simulink model "doa_debug2"  (R2013b) (continued)
== system doa_debug2 == (continued)
      | port_label('output', 1, 'q');
      | disp(['Z^-', num2str(latency)]);
  pipeline91 : Subsystem  AncestorBlock=casper_library_delays/pipeline  mask(pipeline){latency=1}
      mask help:
      | An explicitly laid-out delay line to avoid mapping to SRL16s
      | for use in pipelining to help achieve timing closure.
      mask init:
      | pipeline_init(gcb,'latency',latency);
      settings: AttributesFormatString=Latency = %<latency>
      mask Display:
      | port_label('input', 1, 'd');
      | port_label('output', 1, 'q');
      | disp(['Z^-', num2str(latency)]);
  pipeline92 : Subsystem  AncestorBlock=casper_library_delays/pipeline  mask(pipeline){latency=2}
      mask help:
      | An explicitly laid-out delay line to avoid mapping to SRL16s
      | for use in pipelining to help achieve timing closure.
      mask init:
      | pipeline_init(gcb,'latency',latency);
      settings: AttributesFormatString=Latency = %<latency>
      mask Display:
      | port_label('input', 1, 'd');
      | port_label('output', 1, 'q');
      | disp(['Z^-', num2str(latency)]);
  pipeline97 : Subsystem  AncestorBlock=casper_library_delays/pipeline  mask(pipeline){latency=2}
      mask help:
      | An explicitly laid-out delay line to avoid mapping to SRL16s
      | for use in pipelining to help achieve timing closure.
      mask init:
      | pipeline_init(gcb,'latency',latency);
      settings: AttributesFormatString=Latency = %<latency>
      mask Display:
      | port_label('input', 1, 'd');
      | port_label('output', 1, 'q');
      | disp(['Z^-', num2str(latency)]);
  pipeline98 : Subsystem  AncestorBlock=casper_library_delays/pipeline  mask(pipeline){latency=1}
      mask help:
      | An explicitly laid-out delay line to avoid mapping to SRL16s
      | for use in pipelining to help achieve timing closure.
      mask init:
      | pipeline_init(gcb,'latency',latency);
      settings: AttributesFormatString=Latency = %<latency>
      mask Display:
      | port_label('input', 1, 'd');
      | port_label('output', 1, 'q');
      | disp(['Z^-', num2str(latency)]);
  power{,1..7} (8 alike) : casper_library_misc/power  BitWidth=18  add_latency=2  mult_latency=3
  r11_0 : Subsystem  Tag=xps:bram  AncestorBlock=xps_library/Shared_BRAM  mask{arith_type=Unsigned, addr_width=11, data_width=16, reg_prim_output=off, reg_core_output=off, optimization=Minimum_Area, data_bin_pt=0, init_vals=[0:2^10-1], sample_rate=1}
      mask prompts: arith_type "Output Data Type"; addr_width "Address width"; data_width "Data Width"; reg_prim_output "Register Primitive Output"; reg_core_output "Register Core Output"; data_bin_pt "Data Binary Point"; init_vals "Initial values (simulation only)"; sample_rate "Sample rate"
      mask init:
      | shared_bram_mask;
      mask TypeOptions arith_type: Unsigned | Signed  (2's comp)
      mask TypeOptions data_width: 8 | 16 | 32 | 64 | 128
      mask TypeOptions optimization: Minimum_Area | Low_Power
  r11_1 : Subsystem  Tag=xps:bram  AncestorBlock=xps_library/Shared_BRAM  mask{arith_type=Unsigned, addr_width=11, data_width=16, reg_prim_output=off, reg_core_output=off, optimization=Minimum_Area, data_bin_pt=0, init_vals=[0:2^10-1], sample_rate=1}
      mask prompts: arith_type "Output Data Type"; addr_width "Address width"; data_width "Data Width"; reg_prim_output "Register Primitive Output"; reg_core_output "Register Core Output"; data_bin_pt "Data Binary Point"; init_vals "Initial values (simulation only)"; sample_rate "Sample rate"
      mask init:
      | shared_bram_mask;
      mask TypeOptions arith_type: Unsigned | Signed  (2's comp)
      mask TypeOptions data_width: 8 | 16 | 32 | 64 | 128
      mask TypeOptions optimization: Minimum_Area | Low_Power
  r11_2 : Subsystem  Tag=xps:bram  AncestorBlock=xps_library/Shared_BRAM  mask{arith_type=Unsigned, addr_width=11, data_width=16, reg_prim_output=off, reg_core_output=off, optimization=Minimum_Area, data_bin_pt=0, init_vals=[0:2^10-1], sample_rate=1}
      mask prompts: arith_type "Output Data Type"; addr_width "Address width"; data_width "Data Width"; reg_prim_output "Register Primitive Output"; reg_core_output "Register Core Output"; data_bin_pt "Data Binary Point"; init_vals "Initial values (simulation only)"; sample_rate "Sample rate"
      mask init:
      | shared_bram_mask;
      mask TypeOptions arith_type: Unsigned | Signed  (2's comp)
      mask TypeOptions data_width: 8 | 16 | 32 | 64 | 128
      mask TypeOptions optimization: Minimum_Area | Low_Power
  r11_3 : Subsystem  Tag=xps:bram  AncestorBlock=xps_library/Shared_BRAM  mask{arith_type=Unsigned, addr_width=11, data_width=16, reg_prim_output=off, reg_core_output=off, optimization=Minimum_Area, data_bin_pt=0, init_vals=[0:2^10-1], sample_rate=1}
      mask prompts: arith_type "Output Data Type"; addr_width "Address width"; data_width "Data Width"; reg_prim_output "Register Primitive Output"; reg_core_output "Register Core Output"; data_bin_pt "Data Binary Point"; init_vals "Initial values (simulation only)"; sample_rate "Sample rate"
      mask init:
      | shared_bram_mask;
      mask TypeOptions arith_type: Unsigned | Signed  (2's comp)
      mask TypeOptions data_width: 8 | 16 | 32 | 64 | 128
      mask TypeOptions optimization: Minimum_Area | Low_Power
  r12_0 : Subsystem  Tag=xps:bram  AncestorBlock=xps_library/Shared_BRAM  mask{arith_type=Unsigned, addr_width=11, data_width=16, reg_prim_output=off, reg_core_output=off, optimization=Minimum_Area, data_bin_pt=0, init_vals=[0:2^10-1], sample_rate=1}
      mask prompts: arith_type "Output Data Type"; addr_width "Address width"; data_width "Data Width"; reg_prim_output "Register Primitive Output"; reg_core_output "Register Core Output"; data_bin_pt "Data Binary Point"; init_vals "Initial values (simulation only)"; sample_rate "Sample rate"
      mask init:
      | shared_bram_mask;
      mask TypeOptions arith_type: Unsigned | Signed  (2's comp)
      mask TypeOptions data_width: 8 | 16 | 32 | 64 | 128
      mask TypeOptions optimization: Minimum_Area | Low_Power
  r12_1 : Subsystem  Tag=xps:bram  AncestorBlock=xps_library/Shared_BRAM  mask{arith_type=Unsigned, addr_width=11, data_width=16, reg_prim_output=off, reg_core_output=off, optimization=Minimum_Area, data_bin_pt=0, init_vals=[0:2^10-1], sample_rate=1}
      mask prompts: arith_type "Output Data Type"; addr_width "Address width"; data_width "Data Width"; reg_prim_output "Register Primitive Output"; reg_core_output "Register Core Output"; data_bin_pt "Data Binary Point"; init_vals "Initial values (simulation only)"; sample_rate "Sample rate"
      mask init:
      | shared_bram_mask;
      mask TypeOptions arith_type: Unsigned | Signed  (2's comp)
      mask TypeOptions data_width: 8 | 16 | 32 | 64 | 128
      mask TypeOptions optimization: Minimum_Area | Low_Power
  r12_2 : Subsystem  Tag=xps:bram  AncestorBlock=xps_library/Shared_BRAM  mask{arith_type=Unsigned, addr_width=11, data_width=16, reg_prim_output=off, reg_core_output=off, optimization=Minimum_Area, data_bin_pt=0, init_vals=[0:2^10-1], sample_rate=1}
      mask prompts: arith_type "Output Data Type"; addr_width "Address width"; data_width "Data Width"; reg_prim_output "Register Primitive Output"; reg_core_output "Register Core Output"; data_bin_pt "Data Binary Point"; init_vals "Initial values (simulation only)"; sample_rate "Sample rate"
      mask init:
      | shared_bram_mask;
      mask TypeOptions arith_type: Unsigned | Signed  (2's comp)
      mask TypeOptions data_width: 8 | 16 | 32 | 64 | 128
      mask TypeOptions optimization: Minimum_Area | Low_Power
  r12_3 : Subsystem  Tag=xps:bram  AncestorBlock=xps_library/Shared_BRAM  mask{arith_type=Unsigned, addr_width=11, data_width=16, reg_prim_output=off, reg_core_output=off, optimization=Minimum_Area, data_bin_pt=0, init_vals=[0:2^10-1], sample_rate=1}
      mask prompts: arith_type "Output Data Type"; addr_width "Address width"; data_width "Data Width"; reg_prim_output "Register Primitive Output"; reg_core_output "Register Core Output"; data_bin_pt "Data Binary Point"; init_vals "Initial values (simulation only)"; sample_rate "Sample rate"
      mask init:
      | shared_bram_mask;
      mask TypeOptions arith_type: Unsigned | Signed  (2's comp)
      mask TypeOptions data_width: 8 | 16 | 32 | 64 | 128
      mask TypeOptions optimization: Minimum_Area | Low_Power
  r22_0 : Subsystem  Tag=xps:bram  AncestorBlock=xps_library/Shared_BRAM  mask{arith_type=Unsigned, addr_width=11, data_width=16, reg_prim_output=off, reg_core_output=off, optimization=Minimum_Area, data_bin_pt=0, init_vals=[0:2^10-1], sample_rate=1}
      mask prompts: arith_type "Output Data Type"; addr_width "Address width"; data_width "Data Width"; reg_prim_output "Register Primitive Output"; reg_core_output "Register Core Output"; data_bin_pt "Data Binary Point"; init_vals "Initial values (simulation only)"; sample_rate "Sample rate"
      mask init:
      | shared_bram_mask;
      mask TypeOptions arith_type: Unsigned | Signed  (2's comp)
      mask TypeOptions data_width: 8 | 16 | 32 | 64 | 128
      mask TypeOptions optimization: Minimum_Area | Low_Power
  r22_1 : Subsystem  Tag=xps:bram  AncestorBlock=xps_library/Shared_BRAM  mask{arith_type=Unsigned, addr_width=11, data_width=16, reg_prim_output=off, reg_core_output=off, optimization=Minimum_Area, data_bin_pt=0, init_vals=[0:2^10-1], sample_rate=1}
      mask prompts: arith_type "Output Data Type"; addr_width "Address width"; data_width "Data Width"; reg_prim_output "Register Primitive Output"; reg_core_output "Register Core Output"; data_bin_pt "Data Binary Point"; init_vals "Initial values (simulation only)"; sample_rate "Sample rate"
      mask init:
      | shared_bram_mask;
      mask TypeOptions arith_type: Unsigned | Signed  (2's comp)
      mask TypeOptions data_width: 8 | 16 | 32 | 64 | 128
      mask TypeOptions optimization: Minimum_Area | Low_Power
  r22_2 : Subsystem  Tag=xps:bram  AncestorBlock=xps_library/Shared_BRAM  mask{arith_type=Unsigned, addr_width=11, data_width=16, reg_prim_output=off, reg_core_output=off, optimization=Minimum_Area, data_bin_pt=0, init_vals=[0:2^10-1], sample_rate=1}
      mask prompts: arith_type "Output Data Type"; addr_width "Address width"; data_width "Data Width"; reg_prim_output "Register Primitive Output"; reg_core_output "Register Core Output"; data_bin_pt "Data Binary Point"; init_vals "Initial values (simulation only)"; sample_rate "Sample rate"
      mask init:
      | shared_bram_mask;
      mask TypeOptions arith_type: Unsigned | Signed  (2's comp)
      mask TypeOptions data_width: 8 | 16 | 32 | 64 | 128
      mask TypeOptions optimization: Minimum_Area | Low_Power
  r22_3 : Subsystem  Tag=xps:bram  AncestorBlock=xps_library/Shared_BRAM  mask{arith_type=Unsigned, addr_width=11, data_width=16, reg_prim_output=off, reg_core_output=off, optimization=Minimum_Area, data_bin_pt=0, init_vals=[0:2^10-1], sample_rate=1}
      mask prompts: arith_type "Output Data Type"; addr_width "Address width"; data_width "Data Width"; reg_prim_output "Register Primitive Output"; reg_core_output "Register Core Output"; data_bin_pt "Data Binary Point"; init_vals "Initial values (simulation only)"; sample_rate "Sample rate"
      mask init:
      | shared_bram_mask;
      mask TypeOptions arith_type: Unsigned | Signed  (2's comp)
      mask TypeOptions data_width: 8 | 16 | 32 | 64 | 128
      mask TypeOptions optimization: Minimum_Area | Low_Power
  simple_bram_vacc_0a_0 : Subsystem  AncestorBlock=casper_library_accumulators/simple_bram_vacc  mask(simple_bram_vacc){vec_len=512, arith_type=Unsigned, n_bits=64, bin_pt=35}
      mask prompts: vec_len "Vector length:"; arith_type "Output type:"; n_bits "Bit width (output):"; bin_pt "Binary point (output):"
      mask help: This simple vector accumulator is based on a shift register. It outputs its previous accumulated vector after a new_acc pulse is received.
      mask init:
      | simple_bram_vacc_init(gcb, ...
      |     'vec_len', vec_len, ...
      |     'arith_type', arith_type, ...
      |     'n_bits', n_bits, ...
      |     'bin_pt', bin_pt);
      mask TypeOptions arith_type: Signed | Unsigned
  simple_bram_vacc_0a_1 : Subsystem  AncestorBlock=casper_library_accumulators/simple_bram_vacc  mask(simple_bram_vacc){vec_len=512, arith_type=Unsigned, n_bits=64, bin_pt=35}
      mask prompts: vec_len "Vector length:"; arith_type "Output type:"; n_bits "Bit width (output):"; bin_pt "Binary point (output):"
      mask help: This simple vector accumulator is based on a shift register. It outputs its previous accumulated vector after a new_acc pulse is received.
      mask init:
      | simple_bram_vacc_init(gcb, ...
      |     'vec_len', vec_len, ...
      |     'arith_type', arith_type, ...
      |     'n_bits', n_bits, ...
      |     'bin_pt', bin_pt);
      mask TypeOptions arith_type: Signed | Unsigned
  simple_bram_vacc_0a_2 : Subsystem  AncestorBlock=casper_library_accumulators/simple_bram_vacc  mask(simple_bram_vacc){vec_len=512, arith_type=Unsigned, n_bits=64, bin_pt=35}
      mask prompts: vec_len "Vector length:"; arith_type "Output type:"; n_bits "Bit width (output):"; bin_pt "Binary point (output):"
      mask help: This simple vector accumulator is based on a shift register. It outputs its previous accumulated vector after a new_acc pulse is received.
      mask init:
      | simple_bram_vacc_init(gcb, ...
      |     'vec_len', vec_len, ...
      |     'arith_type', arith_type, ...
      |     'n_bits', n_bits, ...
      |     'bin_pt', bin_pt);
      mask TypeOptions arith_type: Signed | Unsigned
  simple_bram_vacc_0a_3 : Subsystem  AncestorBlock=casper_library_accumulators/simple_bram_vacc  mask(simple_bram_vacc){vec_len=512, arith_type=Unsigned, n_bits=64, bin_pt=35}
      mask prompts: vec_len "Vector length:"; arith_type "Output type:"; n_bits "Bit width (output):"; bin_pt "Binary point (output):"
      mask help: This simple vector accumulator is based on a shift register. It outputs its previous accumulated vector after a new_acc pulse is received.
      mask init:
      | simple_bram_vacc_init(gcb, ...
      |     'vec_len', vec_len, ...
      |     'arith_type', arith_type, ...
      |     'n_bits', n_bits, ...
      |     'bin_pt', bin_pt);
      mask TypeOptions arith_type: Signed | Unsigned
  simple_bram_vacc_0c_0 : Subsystem  AncestorBlock=casper_library_accumulators/simple_bram_vacc  mask(simple_bram_vacc){vec_len=512, arith_type=Unsigned, n_bits=64, bin_pt=35}
      mask prompts: vec_len "Vector length:"; arith_type "Output type:"; n_bits "Bit width (output):"; bin_pt "Binary point (output):"
      mask help: This simple vector accumulator is based on a shift register. It outputs its previous accumulated vector after a new_acc pulse is received.
      mask init:
      | simple_bram_vacc_init(gcb, ...
      |     'vec_len', vec_len, ...
      |     'arith_type', arith_type, ...
      |     'n_bits', n_bits, ...
      |     'bin_pt', bin_pt);
      mask TypeOptions arith_type: Signed | Unsigned
  simple_bram_vacc_0c_1 : Subsystem  AncestorBlock=casper_library_accumulators/simple_bram_vacc  mask(simple_bram_vacc){vec_len=512, arith_type=Unsigned, n_bits=64, bin_pt=35}
      mask prompts: vec_len "Vector length:"; arith_type "Output type:"; n_bits "Bit width (output):"; bin_pt "Binary point (output):"
      mask help: This simple vector accumulator is based on a shift register. It outputs its previous accumulated vector after a new_acc pulse is received.
      mask init:
      | simple_bram_vacc_init(gcb, ...
      |     'vec_len', vec_len, ...
      |     'arith_type', arith_type, ...
      |     'n_bits', n_bits, ...
      |     'bin_pt', bin_pt);
      mask TypeOptions arith_type: Signed | Unsigned
  simple_bram_vacc_0c_2 : Subsystem  AncestorBlock=casper_library_accumulators/simple_bram_vacc  mask(simple_bram_vacc){vec_len=512, arith_type=Unsigned, n_bits=64, bin_pt=35}
      mask prompts: vec_len "Vector length:"; arith_type "Output type:"; n_bits "Bit width (output):"; bin_pt "Binary point (output):"
      mask help: This simple vector accumulator is based on a shift register. It outputs its previous accumulated vector after a new_acc pulse is received.
      mask init:
      | simple_bram_vacc_init(gcb, ...
      |     'vec_len', vec_len, ...
      |     'arith_type', arith_type, ...
      |     'n_bits', n_bits, ...
      |     'bin_pt', bin_pt);
      mask TypeOptions arith_type: Signed | Unsigned
  simple_bram_vacc_0c_3 : Subsystem  AncestorBlock=casper_library_accumulators/simple_bram_vacc  mask(simple_bram_vacc){vec_len=512, arith_type=Unsigned, n_bits=64, bin_pt=35}
      mask prompts: vec_len "Vector length:"; arith_type "Output type:"; n_bits "Bit width (output):"; bin_pt "Binary point (output):"
      mask help: This simple vector accumulator is based on a shift register. It outputs its previous accumulated vector after a new_acc pulse is received.
      mask init:
      | simple_bram_vacc_init(gcb, ...
      |     'vec_len', vec_len, ...
      |     'arith_type', arith_type, ...
      |     'n_bits', n_bits, ...
      |     'bin_pt', bin_pt);
      mask TypeOptions arith_type: Signed | Unsigned
  snap_trig : Subsystem  Tag=xps:sw_reg  AncestorBlock=xps_library/software_register  mask(swreg){io_dir=From Processor, io_delay=0, sample_period=1, names=reg, bitwidths=32, bin_pts=0, arith_types=2, sim_port=off, show_format=off}
      mask prompts: io_dir "I/O direction"; io_delay "I/O delay"; sample_period "Sample period"; names "Bitfield names [msb...lsb]"; bitwidths "Bitfield widths"; bin_pts "Bitfield binary pts"; arith_types "Bitfield types, ufix=0, fix=1, bool=2"; sim_port "Provide sim input/output?"; show_format "Print format string?"
      mask help: A 32-bit software-accessible register. Can be divided into bitfields of varying widths and types using the fields in the block mask.
      mask init:
      | try
      |     swreg_init(gcb)
      | catch ex
      |     disp(sprintf('%s: %s', gcb, ex.message))
      |     errordlg(ex.message)
      | end
      settings: AttributesFormatString=1input
      mask TypeOptions io_dir: To Processor | From Processor
      mask Callback io_dir:
      | mask_enables = get_param(gcb, 'MaskEnables');
      | mask_names = get_param(gcb, 'MaskNames');
      | if strcmp(get_param(gcb, 'io_dir'), 'To Processor'),
      |     mask_enables{ismember(mask_names, 'sample_period')} = 'off';
      | else
      |     mask_enables{ismember(mask_names, 'sample_period')} = 'on';
      | end
      | set_param(gcb, 'MaskEnables', mask_enables);
  sync_gen : Subsystem  AncestorBlock=casper_library_misc/sync_gen  mask(sync_gen){sim_acc_len=1, fft_size=4096, fft_simult_inputs=8, pfb_fir_taps=4, reorder_vec=[2 2 9], scale=1}
      mask prompts: sim_acc_len "Simulation Accumulation Length"; fft_size "FFT Size"; fft_simult_inputs "Simultaneous Inputs (FFT)"; pfb_fir_taps "Taps in pfb_fir (use 1 if no pfb_fir)"; reorder_vec "Reorder Orders (e.g. [2 2 2])"
      mask help:
      | This block computes the sync period based on system parameters as
      | described in Casper memo.  Detailed descritions of the block's
      | variables can be found in the 'Block Documentation' section of
      | the CASPER wiki.
      mask init:
      | sync_gen_init(gcb, ...
      |     'sim_acc_len', sim_acc_len, ...
      |     'fft_size', fft_size, ...
      |     'fft_simult_inputs', fft_simult_inputs,...
      |     'pfb_fir_taps', pfb_fir_taps, ...
      |     'reorder_vec', reorder_vec, ...
      |     'scale', scale);
      settings: AttributesFormatString=sim_sync_period=36864
      mask Help:
      | SyncPeriod=LCM(reorder_vec)*(acc_num)*(pfb_fir_len)*(fft_size/fft_simult_inputs)
      | 
      | See CASPER sync pulse memo for more details.
  wires:
  Constant1/1 -> fft_wideband_real0/2(shift)
  fft_wideband_real0/6(of) -> Terminator1/1
  bus_create/1(bus_out) -> pipeline276/1
  acc_len/1(in_reg) -> pipeline250/1(d)
  cnt_rst/1(in_reg) -> pipeline251/1(d)
  pipeline250/1(q) -> [acc_len]
  pipeline251/1(q) -> Slice7/1, Slice1/1
  Band-Limited White Noise/1 -> asiaa_adc5g0/1(sim_a)
  Constant13/1 -> asiaa_adc5g0/3(sim_sync)
  sync_gen/1(sync_out) -> [sync_out]
  pipeline262/1 -> pfb_fir_real/8(pol1_in7)
  pipeline257/1 -> pfb_fir_real/3(pol1_in2)
  pipeline261/1 -> pfb_fir_real/7(pol1_in6)
  pipeline260/1 -> pfb_fir_real/6(pol1_in5)
  pipeline259/1 -> pfb_fir_real/5(pol1_in4)
  pipeline258/1 -> pfb_fir_real/4(pol1_in3)
  pipeline263/1 -> pfb_fir_real/9(pol1_in8)
  pipeline256/1 -> pfb_fir_real/2(pol1_in1)
  pipeline16/1 -> pfb_fir_real/1(sync)
  pfb_fir_real/1(sync_out) -> Delay15/1
  Delay15/1 -> fft_wideband_real0/1(sync)
  fft_wideband_real0/1(sync_out) -> pipeline275/1
  fft_wideband_real0/2(out00) -> pipeline1/1
  fft_wideband_real0/3(out01) -> pipeline2/1
  fft_wideband_real0/4(out02) -> pipeline3/1
  fft_wideband_real0/5(out03) -> pipeline9/1
  pipeline276/1 -> adcsnap0/1(din)
  [snap_trig] -> adcsnap0/3(trig), adcsnap0/2(we)
  Slice1/1 -> [cnt_rst]
  Shift12/1 -> fft_wideband_real0/5(in02)
  Shift9/1 -> fft_wideband_real0/6(in03)
  Shift11/1 -> fft_wideband_real0/7(in04)
  Shift10/1 -> fft_wideband_real0/8(in05)
  Shift8/1 -> fft_wideband_real0/9(in06)
  Shift7/1 -> fft_wideband_real0/10(in07)
  Shift13/1 -> fft_wideband_real0/4(in01)
  Shift15/1 -> fft_wideband_real0/3(in00)
  pfb_fir_real/2(pol1_out1) -> Shift15/1
  pfb_fir_real/3(pol1_out2) -> Shift13/1
  pfb_fir_real/4(pol1_out3) -> Shift12/1
  pfb_fir_real/5(pol1_out4) -> Shift9/1
  pfb_fir_real/6(pol1_out5) -> Shift11/1
  pfb_fir_real/7(pol1_out6) -> Shift10/1
  pfb_fir_real/8(pol1_out7) -> Shift8/1
  pfb_fir_real/9(pol1_out8) -> Shift7/1
  Band-Limited White Noise2/1 -> asiaa_adc5g0/2(sim_c)
  asiaa_adc5g0/17(sync_out) -> Terminator/1
  asiaa_adc5g0/1(a0) -> conv_bus0/1(i0)
  asiaa_adc5g0/2(a1) -> conv_bus0/2(i1)
  asiaa_adc5g0/3(a2) -> conv_bus0/3(i2)
  asiaa_adc5g0/4(a3) -> conv_bus0/4(i3)
  asiaa_adc5g0/5(a4) -> conv_bus0/5(i4)
  asiaa_adc5g0/6(a5) -> conv_bus0/6(i5)
  asiaa_adc5g0/7(a6) -> conv_bus0/7(i6)
  asiaa_adc5g0/8(a7) -> conv_bus0/8(i7)
  conv_bus0/7(q6) -> bus_create/7(in7), pipeline262/1
  conv_bus0/5(q4) -> bus_create/5(in5), pipeline260/1
  conv_bus0/4(q3) -> bus_create/4(in4), pipeline259/1
  conv_bus0/3(q2) -> bus_create/3(in3), pipeline258/1
  conv_bus0/2(q1) -> bus_create/2(in2), pipeline257/1
  conv_bus0/1(q0) -> bus_create/1(in1), pipeline256/1
  bus_create1/1(bus_out) -> pipeline4/1
  pipeline4/1 -> adcsnap1/1(din)
  [snap_trig] -> adcsnap1/3(trig), adcsnap1/2(we)
  conv_bus0/8(q7) -> bus_create/8(in8), pipeline263/1
  [sync_out] -> pipeline16/1
  pipeline72/1(q) -> acc_cntrl0/2(sync)
  [cnt_rst] -> acc_cntrl0/3(rst)
  [acc_len] -> acc_cntrl0/1(acc_len)
  power/1 -> Delay32/1
  power1/1 -> Delay34/1
  power2/1 -> Delay35/1
  power3/1 -> Delay36/1
  dout_0a_0/1(data_out) -> Terminator9/1
  Reinterpret31/1 -> dout_0a_0/2(data_in)
  simple_bram_vacc_0a_0/1(dout) -> pipeline74/1(d)
  simple_bram_vacc_0a_0/2(valid) -> pipeline56/1(d)
  pipeline73/1(q) -> simple_bram_vacc_0a_0/2(din)
  pipeline59/1(q) -> pipeline57/1(d)
  pipeline74/1(q) -> Reinterpret31/1
  pipeline56/1(q) -> Counter1/2, dout_0a_0/3(we)
  pipeline57/1(q) -> Counter1/1
  pipeline58/1(q) -> simple_bram_vacc_0a_0/1(new_acc)
  dout_0a_1/1(data_out) -> Terminator6/1
  Reinterpret24/1 -> dout_0a_1/2(data_in)
  simple_bram_vacc_0a_1/1(dout) -> pipeline61/1(d)
  simple_bram_vacc_0a_1/2(valid) -> pipeline62/1(d)
  pipeline60/1(q) -> simple_bram_vacc_0a_1/2(din)
  pipeline61/1(q) -> Reinterpret24/1
  pipeline62/1(q) -> dout_0a_1/3(we)
  pipeline63/1(q) -> simple_bram_vacc_0a_1/1(new_acc)
  dout_0a_2/1(data_out) -> Terminator7/1
  Reinterpret25/1 -> dout_0a_2/2(data_in)
  simple_bram_vacc_0a_2/1(dout) -> pipeline65/1(d)
  simple_bram_vacc_0a_2/2(valid) -> pipeline66/1(d)
  pipeline64/1(q) -> simple_bram_vacc_0a_2/2(din)
  pipeline65/1(q) -> Reinterpret25/1
  pipeline66/1(q) -> dout_0a_2/3(we)
  pipeline67/1(q) -> simple_bram_vacc_0a_2/1(new_acc)
  dout_0a_3/1(data_out) -> Terminator8/1
  Reinterpret26/1 -> dout_0a_3/2(data_in)
  simple_bram_vacc_0a_3/1(dout) -> pipeline69/1(d)
  simple_bram_vacc_0a_3/2(valid) -> pipeline70/1(d)
  pipeline68/1(q) -> simple_bram_vacc_0a_3/2(din)
  pipeline69/1(q) -> Reinterpret26/1
  pipeline70/1(q) -> dout_0a_3/3(we)
  pipeline71/1(q) -> simple_bram_vacc_0a_3/1(new_acc)
  Delay32/1 -> pipeline73/1(d)
  Delay34/1 -> pipeline60/1(d)
  Delay35/1 -> pipeline64/1(d)
  Delay36/1 -> pipeline68/1(d)
  Counter1/1 -> dout_0a_1/1(addr), dout_0a_2/1(addr), dout_0a_3/1(addr), dout_0a_0/1(addr)
  acc_cntrl0/1(new_acc) -> pipeline63/1(d), pipeline71/1(d), pipeline67/1(d), pipeline58/1(d), pipeline59/1(d)
  pipeline275/1 -> pipeline72/1(d), pipeline33/1
  pipeline1/1 -> power/1, pipeline34/1
  pipeline2/1 -> pipeline35/1, power1/1
  pipeline3/1 -> pipeline36/1, power2/1
  pipeline9/1 -> pipeline37/1, power3/1
  Constant10/1 -> fft_wideband_real1/2(shift)
  fft_wideband_real1/6(of) -> Terminator5/1
  pipeline18/1 -> pfb_fir_real1/8(pol1_in7)
  pipeline11/1 -> pfb_fir_real1/3(pol1_in2)
  pipeline17/1 -> pfb_fir_real1/7(pol1_in6)
  pipeline15/1 -> pfb_fir_real1/6(pol1_in5)
  pipeline14/1 -> pfb_fir_real1/5(pol1_in4)
  pipeline12/1 -> pfb_fir_real1/4(pol1_in3)
  pipeline19/1 -> pfb_fir_real1/9(pol1_in8)
  pipeline10/1 -> pfb_fir_real1/2(pol1_in1)
  pipeline6/1 -> pfb_fir_real1/1(sync)
  pfb_fir_real1/1(sync_out) -> Delay3/1
  Delay3/1 -> fft_wideband_real1/1(sync)
  fft_wideband_real1/1(sync_out) -> pipeline20/1
  fft_wideband_real1/2(out00) -> pipeline5/1
  fft_wideband_real1/3(out01) -> pipeline7/1
  fft_wideband_real1/4(out02) -> pipeline21/1
  fft_wideband_real1/5(out03) -> pipeline93/1
  Shift3/1 -> fft_wideband_real1/5(in02)
  Shift16/1 -> fft_wideband_real1/6(in03)
  Shift2/1 -> fft_wideband_real1/7(in04)
  Shift1/1 -> fft_wideband_real1/8(in05)
  Shift14/1 -> fft_wideband_real1/9(in06)
  Shift6/1 -> fft_wideband_real1/10(in07)
  Shift4/1 -> fft_wideband_real1/4(in01)
  Shift5/1 -> fft_wideband_real1/3(in00)
  pfb_fir_real1/2(pol1_out1) -> Shift5/1
  pfb_fir_real1/3(pol1_out2) -> Shift4/1
  pfb_fir_real1/4(pol1_out3) -> Shift3/1
  pfb_fir_real1/5(pol1_out4) -> Shift16/1
  pfb_fir_real1/6(pol1_out5) -> Shift2/1
  pfb_fir_real1/7(pol1_out6) -> Shift1/1
  pfb_fir_real1/8(pol1_out7) -> Shift14/1
  pfb_fir_real1/9(pol1_out8) -> Shift6/1
  conv_bus1/7(q6) -> pipeline18/1, bus_create1/7(in7)
  conv_bus1/6(q5) -> bus_create1/6(in6), pipeline17/1
  conv_bus1/5(q4) -> bus_create1/5(in5), pipeline15/1
  conv_bus1/4(q3) -> bus_create1/4(in4), pipeline14/1
  conv_bus1/3(q2) -> bus_create1/3(in3), pipeline12/1
  conv_bus1/2(q1) -> bus_create1/2(in2), pipeline11/1
  conv_bus1/1(q0) -> bus_create1/1(in1), pipeline10/1
  conv_bus1/8(q7) -> bus_create1/8(in8), pipeline19/1
  [sync_out] -> pipeline6/1
  pipeline90/1(q) -> acc_cntrl1/2(sync)
  [cnt_rst] -> acc_cntrl1/3(rst)
  [acc_len] -> acc_cntrl1/1(acc_len)
  power4/1 -> Delay7/1
  power5/1 -> Delay8/1
  power6/1 -> Delay9/1
  power7/1 -> Delay10/1
  dout_0c_0/1(data_out) -> Terminator13/1
  Reinterpret4/1 -> dout_0c_0/2(data_in)
  simple_bram_vacc_0c_0/1(dout) -> pipeline92/1(d)
  simple_bram_vacc_0c_0/2(valid) -> pipeline22/1(d)
  pipeline91/1(q) -> simple_bram_vacc_0c_0/2(din)
  pipeline77/1(q) -> pipeline75/1(d)
  pipeline92/1(q) -> Reinterpret4/1
  pipeline22/1(q) -> dout_0c_0/3(we), Counter2/2
  pipeline75/1(q) -> Counter2/1
  pipeline76/1(q) -> simple_bram_vacc_0c_0/1(new_acc)
  dout_0c_1/1(data_out) -> Terminator10/1
  Reinterpret1/1 -> dout_0c_1/2(data_in)
  simple_bram_vacc_0c_1/1(dout) -> pipeline79/1(d)
  simple_bram_vacc_0c_1/2(valid) -> pipeline80/1(d)
  pipeline78/1(q) -> simple_bram_vacc_0c_1/2(din)
  pipeline79/1(q) -> Reinterpret1/1
  pipeline80/1(q) -> dout_0c_1/3(we)
  pipeline81/1(q) -> simple_bram_vacc_0c_1/1(new_acc)
  dout_0c_2/1(data_out) -> Terminator11/1
  Reinterpret2/1 -> dout_0c_2/2(data_in)
  simple_bram_vacc_0c_2/1(dout) -> pipeline83/1(d)
  simple_bram_vacc_0c_2/2(valid) -> pipeline84/1(d)
  pipeline82/1(q) -> simple_bram_vacc_0c_2/2(din)
  pipeline83/1(q) -> Reinterpret2/1
  pipeline84/1(q) -> dout_0c_2/3(we)
  pipeline85/1(q) -> simple_bram_vacc_0c_2/1(new_acc)
  dout_0c_3/1(data_out) -> Terminator12/1
  Reinterpret3/1 -> dout_0c_3/2(data_in)
  simple_bram_vacc_0c_3/1(dout) -> pipeline87/1(d)
  simple_bram_vacc_0c_3/2(valid) -> pipeline88/1(d)
  pipeline86/1(q) -> simple_bram_vacc_0c_3/2(din)
  pipeline87/1(q) -> Reinterpret3/1
  pipeline88/1(q) -> dout_0c_3/3(we)
  pipeline89/1(q) -> simple_bram_vacc_0c_3/1(new_acc)
  Delay7/1 -> pipeline91/1(d)
  Delay8/1 -> pipeline78/1(d)
  Delay9/1 -> pipeline82/1(d)
  Delay10/1 -> pipeline86/1(d)
  Counter2/1 -> dout_0c_0/1(addr), dout_0c_1/1(addr), dout_0c_2/1(addr), dout_0c_3/1(addr)
  acc_cntrl1/1(new_acc) -> pipeline77/1(d), pipeline76/1(d), pipeline85/1(d), pipeline89/1(d), pipeline81/1(d)
  pipeline20/1 -> pipeline90/1(d)
  pipeline5/1 -> pipeline39/1, power4/1
  pipeline7/1 -> power5/1, pipeline40/1
  pipeline21/1 -> pipeline41/1, power6/1
  pipeline93/1 -> pipeline42/1, power7/1
  asiaa_adc5g0/12(c3) -> conv_bus1/4(i3)
  asiaa_adc5g0/14(c5) -> conv_bus1/6(i5)
  asiaa_adc5g0/10(c1) -> conv_bus1/2(i1)
  asiaa_adc5g0/13(c4) -> conv_bus1/5(i4)
  asiaa_adc5g0/9(c0) -> conv_bus1/1(i0)
  asiaa_adc5g0/15(c6) -> conv_bus1/7(i6)
  asiaa_adc5g0/16(c7) -> conv_bus1/8(i7)
  asiaa_adc5g0/11(c2) -> conv_bus1/3(i2)
  conv_bus0/6(q5) -> bus_create/6(in6), pipeline261/1
  c_to_ri/1 -> Black Box4/1
  c_to_ri/2 -> Black Box4/2
  c_to_ri1/1 -> Black Box4/3
  c_to_ri1/2 -> Black Box4/4
  c_to_ri2/1 -> Black Box1/1
  c_to_ri2/2 -> Black Box1/2
  c_to_ri3/1 -> Black Box1/3
  c_to_ri3/2 -> Black Box1/4
  c_to_ri4/1 -> Black Box2/1
  c_to_ri4/2 -> Black Box2/2
  c_to_ri5/1 -> Black Box2/3
  c_to_ri5/2 -> Black Box2/4
  c_to_ri6/1 -> Black Box3/1
  c_to_ri6/2 -> Black Box3/2
  c_to_ri7/1 -> Black Box3/3
  c_to_ri7/2 -> Black Box3/4
  [adc0_ch0] -> c_to_ri/1
  [adc1_ch0] -> c_to_ri1/1
  [adc0_ch1] -> c_to_ri2/1
  [adc1_ch1] -> c_to_ri3/1
  [adc0_ch2] -> c_to_ri4/1
  [adc1_ch2] -> c_to_ri5/1
  [adc0_ch3] -> c_to_ri6/1
  [adc1_ch3] -> c_to_ri7/1
  doa_en/1(in_reg) -> pipeline31/1(d)
  pipeline31/1(q) -> Slice3/1, Slice2/1
  Slice2/1 -> [doa_en]
  [sync_fft] -> acc_cntrl2/2(sync)
  doa_acc/1(in_reg) -> pipeline32/1(d)
  pipeline32/1(q) -> [doa_acc]
  [doa_en] -> Inverter/1
  Inverter/1 -> acc_cntrl2/3(rst), Register/2
  [doa_acc] -> acc_cntrl2/1(acc_len)
  acc_cntrl2/1(new_acc) -> Reinterpret5/1
  [sync_fft] -> Register/3, Register/1
  Register/1 -> Reinterpret/1
  Black Box4/1 -> pipeline43/1
  Black Box4/2 -> pipeline44/1
  Black Box4/3 -> pipeline45/1
  Black Box1/1 -> pipeline46/1
  Black Box1/2 -> pipeline47/1
  Black Box1/3 -> pipeline48/1
  Black Box2/1 -> pipeline49/1
  Black Box2/2 -> pipeline50/1
  Black Box2/3 -> pipeline51/1
  Black Box3/1 -> pipeline52/1
  Black Box3/2 -> pipeline53/1
  Black Box3/3 -> pipeline54/1
  Black Box4/4 -> pipeline55/1
  Black Box1/4 -> pipeline94/1
  Black Box2/4 -> pipeline95/1
  Black Box3/4 -> pipeline96/1
  pipeline55/1 -> Convert1/1
  Counter/1 -> r22_0/1(addr), r12_0/1(addr), r11_0/1(addr)
  pipeline43/1 -> pipeline8/1(d)
  pipeline94/1 -> Convert2/1
  pipeline46/1 -> pipeline26/1(d)
  pipeline44/1 -> pipeline13/1(d)
  pipeline45/1 -> pipeline23/1(d)
  pipeline47/1 -> pipeline24/1(d)
  pipeline48/1 -> pipeline25/1(d)
  pipeline95/1 -> Convert3/1
  Counter4/1 -> r12_2/1(addr), r22_2/1(addr), r11_2/1(addr)
  pipeline49/1 -> pipeline29/1(d)
  pipeline50/1 -> pipeline27/1(d)
  pipeline51/1 -> pipeline28/1(d)
  pipeline96/1 -> Convert4/1
  Counter5/1 -> r12_3/1(addr), r22_3/1(addr), r11_3/1(addr)
  pipeline52/1 -> pipeline97/1(d)
  pipeline53/1 -> pipeline30/1(d)
  pipeline54/1 -> pipeline38/1(d)
  Slice3/1 -> [doa_mem_rst]
  Reinterpret/1 -> Logical/1, Black Box4/5, Black Box1/5, Black Box3/5, Black Box2/5
  Logical/1 -> Black Box4/6, Black Box1/6, Black Box3/6, Black Box2/6
  Reinterpret6/1 -> Subsystem2/3(r11_in)
  Reinterpret7/1 -> Subsystem2/4(r22_in)
  Reinterpret8/1 -> Subsystem2/5(r12_in)
  Reinterpret5/1 -> Logical/2
  pipeline33/1 -> Subsystem4/2(sync_in)
  pipeline34/1 -> Subsystem4/3(din0)
  pipeline35/1 -> Subsystem4/4(din1)
  pipeline36/1 -> Subsystem4/5(din2)
  pipeline37/1 -> Subsystem4/6(din3)
  pipeline39/1 -> Subsystem1/3(din0)
  pipeline40/1 -> Subsystem1/4(din1)
  pipeline41/1 -> Subsystem1/5(din2)
  pipeline42/1 -> Subsystem1/6(din3)
  Subsystem1/2(dout0) -> [adc1_ch0]
  Subsystem1/3(dout1) -> [adc1_ch1]
  Subsystem1/4(dout2) -> [adc1_ch2]
  Subsystem1/5(dout3) -> [adc1_ch3]
  Subsystem4/5(dout3) -> [adc0_ch3]
  Subsystem4/4(dout2) -> [adc0_ch2]
  Subsystem4/1(sync_out) -> [sync_fft]
  Subsystem4/2(dout0) -> [adc0_ch0]
  Subsystem4/3(dout1) -> [adc0_ch1]
  doa_gain/1(in_reg) -> pipeline127/1(d)
  pipeline127/1(q) -> Slice4/1
  Slice4/1 -> [doa_gain]
  [doa_gain] -> Subsystem4/1(gain)
  [doa_gain] -> Subsystem1/1(gain)
  Constant/1 -> Subsystem1/2(sync_in)
  Subsystem1/1(sync_out) -> Terminator2/1
  snap_trig/1(in_reg) -> pipeline128/1(d)
  pipeline128/1(q) -> Slice5/1
  Slice5/1 -> [snap_trig]
  doa_gain1/1(in_reg) -> pipeline98/1(d)
  pipeline98/1(q) -> Slice6/1
  Slice6/1 -> Reinterpret18/1
  Reinterpret18/1 -> [doa_gain1]
  [doa_gain1] -> Subsystem2/1(gain)
  pipeline8/1(q) -> Reinterpret6/1
  pipeline13/1(q) -> Reinterpret7/1
  pipeline23/1(q) -> Reinterpret8/1
  Convert1/1 -> Subsystem2/2(din_valid)
  Subsystem2/2(r11_out) -> pipeline99/1
  Subsystem2/3(r22_out) -> pipeline100/1
  Subsystem2/4(r12_out) -> pipeline101/1
  Subsystem2/1(dout_valid) -> pipeline/1
  Reinterpret9/1 -> Subsystem3/3(r11_in)
  Reinterpret10/1 -> Subsystem3/4(r22_in)
  Reinterpret11/1 -> Subsystem3/5(r12_in)
  [doa_gain1] -> Subsystem3/1(gain)
  pipeline26/1(q) -> Reinterpret9/1
  pipeline24/1(q) -> Reinterpret10/1
  pipeline25/1(q) -> Reinterpret11/1
  Convert2/1 -> Subsystem3/2(din_valid)
  Subsystem3/2(r11_out) -> pipeline103/1
  Subsystem3/3(r22_out) -> pipeline104/1
  Subsystem3/4(r12_out) -> pipeline105/1
  Subsystem3/1(dout_valid) -> pipeline102/1
  Counter3/1 -> r11_1/1(addr), r22_1/1(addr), r12_1/1(addr)
  Reinterpret14/1 -> Subsystem5/3(r11_in)
  Reinterpret12/1 -> Subsystem5/4(r22_in)
  Reinterpret13/1 -> Subsystem5/5(r12_in)
  [doa_gain1] -> Subsystem5/1(gain)
  pipeline29/1(q) -> Reinterpret14/1
  pipeline27/1(q) -> Reinterpret12/1
  pipeline28/1(q) -> Reinterpret13/1
  Convert3/1 -> Subsystem5/2(din_valid)
  Subsystem5/1(dout_valid) -> pipeline106/1
  Subsystem5/2(r11_out) -> pipeline107/1
  Subsystem5/4(r12_out) -> pipeline109/1
  Subsystem5/3(r22_out) -> pipeline108/1
  Reinterpret17/1 -> Subsystem6/3(r11_in)
  Reinterpret15/1 -> Subsystem6/4(r22_in)
  Reinterpret16/1 -> Subsystem6/5(r12_in)
  [doa_gain1] -> Subsystem6/1(gain)
  pipeline97/1(q) -> Reinterpret17/1
  pipeline30/1(q) -> Reinterpret15/1
  pipeline38/1(q) -> Reinterpret16/1
  Convert4/1 -> Subsystem6/2(din_valid)
  Subsystem6/1(dout_valid) -> pipeline110/1
  Subsystem6/4(r12_out) -> pipeline113/1
  Subsystem6/3(r22_out) -> pipeline112/1
  Subsystem6/2(r11_out) -> pipeline111/1
  pipeline/1 -> [val0], r11_0/3(we), r22_0/3(we), r12_0/3(we), Counter/1
  pipeline99/1 -> [r11_0], r11_0/2(data_in)
  pipeline100/1 -> [r22_0], r22_0/2(data_in)
  pipeline101/1 -> [r12_0], r12_0/2(data_in)
  pipeline102/1 -> [val1], r12_1/3(we), r22_1/3(we), r11_1/3(we), Counter3/1
  pipeline103/1 -> [r11_1], r11_1/2(data_in)
  pipeline104/1 -> [r22_1], r22_1/2(data_in)
  pipeline105/1 -> [r12_1], r12_1/2(data_in)
  pipeline106/1 -> [val2], r22_2/3(we), r12_2/3(we), r11_2/3(we), Counter4/1
  pipeline107/1 -> [r11_2], r11_2/2(data_in)
  pipeline108/1 -> [r22_2], r22_2/2(data_in)
  pipeline109/1 -> [r12_2], r12_2/2(data_in)
  pipeline113/1 -> [r12_3], r12_3/2(data_in)
  pipeline110/1 -> [val3], r22_3/3(we), r12_3/3(we), r11_3/3(we), Counter5/1
  pipeline112/1 -> [r22_3], r22_3/2(data_in)
  pipeline111/1 -> [r11_3], r11_3/2(data_in)
  [val1] -> Black Box5/5
  [r11_1] -> Black Box5/2
  [r22_1] -> Black Box5/3
  [r12_1] -> Black Box5/4
  [val2] -> Black Box6/5
  [r11_2] -> Black Box6/2
  [r22_2] -> Black Box6/3
  [r12_2] -> Black Box6/4
  [val3] -> Black Box7/5
  [r11_3] -> Black Box7/2
  [r22_3] -> Black Box7/3
  [r12_3] -> Black Box7/4
  Black Box12/3 -> pipeline114/1
  Black Box12/5 -> pipeline115/1
  Black Box12/7 -> Convert8/1
  Black Box5/3 -> pipeline117/1
  Black Box5/5 -> pipeline118/1
  Black Box6/3 -> pipeline120/1
  Black Box6/5 -> pipeline121/1
  Black Box7/3 -> pipeline123/1
  Black Box7/5 -> pipeline124/1
  Black Box5/4 -> Terminator4/1
  Black Box6/4 -> Terminator14/1
  Black Box7/4 -> Terminator15/1
  Slice7/1 -> [atan_rst]
  [atan_rst] -> Black Box9/1
  Black Box/1 -> pipeline133/1
  Black Box9/3 -> pipeline141/1
  Concat6/1 -> phase2/2(data_in)
  Black Box10/3 -> pipeline144/1
  Concat7/1 -> phase3/2(data_in)
  [atan_rst] -> Black Box10/1
  Black Box12/1 -> Concat/1
  Black Box12/2 -> Concat/2
  Concat/1 -> l0/2(data_in)
  Black Box5/7 -> Convert7/1
  Concat1/1 -> l1/2(data_in)
  Black Box5/1 -> Concat1/1
  Black Box5/2 -> Concat1/2
  Black Box6/7 -> Convert6/1
  Concat2/1 -> l2/2(data_in)
  Black Box6/1 -> Concat2/1
  Black Box6/2 -> Concat2/2
  Black Box7/7 -> Convert5/1
  Concat3/1 -> l3/2(data_in)
  Black Box7/1 -> Concat3/1
  Black Box7/2 -> Concat3/2
  Convert5/1 -> l3/3(we), pipeline125/1
  Convert6/1 -> l2/3(we), pipeline122/1
  Convert7/1 -> l1/3(we), pipeline119/1
  Convert8/1 -> pipeline116/1, l0/3(we)
  capture/1(in_reg) -> pipeline126/1(d)
  pipeline126/1(q) -> [capture]
  Black Box6/6 -> pipeline131/1, l2/1(addr)
  Black Box7/6 -> l3/1(addr), pipeline132/1
  [r11_0] -> Black Box12/2
  [r22_0] -> Black Box12/3
  [r12_0] -> Black Box12/4
  [val0] -> Black Box12/5
  Black Box12/6 -> pipeline129/1, l0/1(addr)
  pipeline114/1 -> Black Box/3
  pipeline115/1 -> Black Box/4
  pipeline116/1 -> Black Box/5
  pipeline129/1 -> Black Box/2
  [atan_rst] -> Black Box/1
  [cnt_rst] -> Black Box12/1
  Black Box/3 -> pipeline135/1
  Black Box/2 -> pipeline134/1
  Constant2/1 -> Concat4/1
  Concat4/1 -> phase0/2(data_in)
  Black Box5/6 -> l1/1(addr), pipeline130/1
  pipeline119/1 -> Black Box8/5
  pipeline118/1 -> Black Box8/4
  pipeline130/1 -> Black Box8/2
  pipeline117/1 -> Black Box8/3
  Black Box8/3 -> pipeline138/1
  Constant3/1 -> Concat5/1
  Concat5/1 -> phase1/2(data_in)
  [cnt_rst] -> Black Box5/1
  [atan_rst] -> Black Box8/1
  [cnt_rst] -> Black Box6/1
  pipeline122/1 -> Black Box9/5
  pipeline121/1 -> Black Box9/4
  pipeline120/1 -> Black Box9/3
  pipeline131/1 -> Black Box9/2
  Constant4/1 -> Concat6/1
  pipeline132/1 -> Black Box10/2
  pipeline123/1 -> Black Box10/3
  pipeline124/1 -> Black Box10/4
  pipeline125/1 -> Black Box10/5
  Constant5/1 -> Concat7/1
  Convert10/1 -> Logical1/2, phase0/3(we)
  [cnt_rst] -> Black Box7/1
  [capture] -> Relational/1
  Relational/1 -> Logical1/1
  Logical1/1 -> freeze_cntr/1
  freeze_cntr/1 -> phase0_cont/1(addr)
  freeze_cntr/2 -> phase0_cont/3(we)
  Black Box8/1 -> pipeline136/1
  Black Box8/2 -> pipeline137/1
  Convert9/1 -> phase1/3(we), Logical2/2
  [capture] -> Relational1/1
  Relational1/1 -> Logical2/1
  Logical2/1 -> freeze_cntr1/1
  freeze_cntr1/1 -> phase1_cont/1(addr)
  freeze_cntr1/2 -> phase1_cont/3(we)
  Black Box9/1 -> pipeline139/1
  Black Box9/2 -> pipeline140/1
  Convert11/1 -> Logical3/2, phase2/3(we)
  [capture] -> Relational2/1
  Relational2/1 -> Logical3/1
  Logical3/1 -> freeze_cntr2/1
  freeze_cntr2/1 -> phase2_cont/1(addr)
  freeze_cntr2/2 -> phase2_cont/3(we)
  Black Box10/1 -> pipeline142/1
  Black Box10/2 -> pipeline143/1
  Convert12/1 -> phase3/3(we), Logical4/2
  [capture] -> Relational3/1
  Relational3/1 -> Logical4/1
  Logical4/1 -> freeze_cntr3/1
  freeze_cntr3/1 -> phase3_cont/1(addr)
  freeze_cntr3/2 -> phase3_cont/3(we)
  [doa_mem_rst] -> freeze_cntr/2
  [doa_mem_rst] -> freeze_cntr1/2
  [doa_mem_rst] -> freeze_cntr2/2
  [doa_mem_rst] -> freeze_cntr3/2
  pipeline133/1 -> Relational/2, phase0/1(addr)
  pipeline134/1 -> phase0_cont/2(data_in), Concat4/2
  pipeline135/1 -> Convert10/1
  pipeline136/1 -> Relational1/2, phase1/1(addr)
  pipeline137/1 -> phase1_cont/2(data_in), Concat5/2
  pipeline138/1 -> Convert9/1
  pipeline139/1 -> Relational2/2, phase2/1(addr)
  pipeline140/1 -> phase2_cont/2(data_in), Concat6/2
  pipeline141/1 -> Convert11/1
  pipeline142/1 -> Relational3/2, phase3/1(addr)
  pipeline143/1 -> phase3_cont/2(data_in), Concat7/2
  pipeline144/1 -> Convert12/1
